simulink model "Complete_Model"  (R2023a)
settings: Solver=VariableStepAuto  StartTime=0.0  StopTime=22  MinStep=5e-5  RelTol=1e-3
values from neighbouring files (symbols this model uses):
  Cbus = 80e-3*n_wt_agg*u^2    (= 1.33333333333)    [param.m]
  D = 1e-3    (= 0.001)    [param.m]
  f = 50    [param.m]
  Fs = Vs * sqrt(2/3)/(2*pi*f)    [param.m]
  fsw = 4e3    (= 4000)    [param.m]
  Idr_rated = sqrt(2)*Is    [param.m]
  J = 127*n_wt_agg    (= 19050)    [param.m]
  ki_id = (wni^2)*Lr*sigma    (= 3.27733001332)    [param.m]
  ki_idg = (wnig^2)*Lg    (= 0.263189450696)    [param.m]
  ki_iq = ki_id    (= 3.27733001332)    [param.m]
  ki_iqg = ki_idg    (= 0.263189450696)    [param.m]
  ki_v = -300000*n_wt_agg    (= -45000000)    [param.m]
  Kopt = ((0.5*rho*pi*(Radius^5)*Cp_max)/(lambda_opt^3))    (= 296454.198186)    [param.m]
  kp_id = (2*wni*sigma*Lr) - Rr    (= 0.00384733333333)    [param.m]
  kp_idg = ((2*wnig*Lg)-Rg)    (= 0.00167538274858)    [param.m]
  kp_iq = kp_id    (= 0.00384733333333)    [param.m]
  kp_iqg = kp_idg    (= 0.00167538274858)    [param.m]
  kp_v = -1000*n_wt_agg    (= -150000)    [param.m]
  Kpg = 1/(1.5*Vr*sqrt(2/3))    [param.m]
  Kqg = -Kpg    [param.m]
  lambda_opt = 7.2    [param.m]
  Lg = 400e-6 /n_wt_agg    (= 2.66666666667e-06)    [param.m]
  Lm = 2.5e-3 /n_wt_agg    (= 1.66666666667e-05)    [param.m]
  Lr = Lm + Lsi    (= 1.72466666667e-05)    [param.m]
  Ls = Lm + Lsi    (= 1.72466666667e-05)    [param.m]
  Lsi = 0.087e-3 /n_wt_agg    (= 5.8e-07)    [param.m]
  N = 100    [param.m]
  n_wt_agg = 150    [param.m]
  p = 2    [param.m]
  Ps = 2e6*n_wt_agg    (= 300000000)    [param.m]
  Radius = 42    [param.m]
  Rg = 20e-6 /n_wt_agg    (= 1.33333333333e-07)    [param.m]
  rho = 1.225    [param.m]
  Rr = 2.9e-3 /n_wt_agg    (= 1.93333333333e-05)    [param.m]
  Rs = 2.6e-3 /n_wt_agg    (= 1.73333333333e-05)    [param.m]
  sigma = 1 - Lm^2 / (Ls*Lr)    (= 0.0661284179513)    [param.m]
  smax = 1/3    (= 0.333333333333)    [param.m]
  tab_lambda = [0.1:0.01:11.8]    [param.m]
  Vbus = 1150/u    (= 3450)    [param.m]
  Vs = 690    [param.m]
undefined symbols (not defined in the file or in its folder): Cp, D1, Delta, DiscIntNeverNeededParam, magnitude
libraries: spsSecondOrderFilterLib x133, spsThreePhaseVIMeasurementLib x58, spsPower3phInstantaneousLib x52, simulink x41, spsThreePhaseTransformerTwoWindingsLib x27, spsUniversalBridgeLib x22, spsPWMGenerator2LevelLib x22, spsSeriesRLCBranchLib x19, spsFirstOrderFilterLib x18, spsThreePhasePISectionLineLib x17, spsGroundLib x17, spsThreePhaseSeriesRLCBranchLib x17
(part 5 of 15)

== system Complete_Model/HVDC Link/Grid side control ==
  note: DC Voltage Control
  note: Reactive Power Control
  Vdc_mes : Inport
  Vdc_ref : Inport  Port=2
  Q_grid : Inport  Port=3
  Qref_grid : Inport  Port=4
  Clarke - Park Transform1 : eeTransforms/Park Transform  AxisAlignment=D-axis  PowerInvariant=off
      settings: LibrarySourceBlock=|
      | ee_sl_lib/Mathematical
      | Transforms/Park Transform
  Clarke - Park Transform2 : eeTransforms/Park Transform  AxisAlignment=D-axis  PowerInvariant=off
      settings: LibrarySourceBlock=|
      | ee_sl_lib/Mathematical
      | Transforms/Park Transform
  Clarke - Park Transform3 : eeTransforms/Park Transform  AxisAlignment=D-axis  PowerInvariant=off
      settings: LibrarySourceBlock=|
      | ee_sl_lib/Mathematical
      | Transforms/Park Transform
  Constant : Constant  Value=0
  Constant1 : Constant  Value=0
  Constant4 : Constant  Value=0
  Demux{1..5,7} (6 alike) : Demux  Outputs=2
      Demux2 settings: DisplayOption=none
      Demux3 settings: DisplayOption=none
  Display5 : Display
  Display6 : Display
  Divide : Product  Inputs=*/
  First-Order Filter{1,4..6} (4 alike) : spsFirstOrderFilterLib/First-Order Filter  FilterType=Lowpass  Tc=0.015915  Ts=7.4074e-05  Initialize=on  Vac_Init=[0, 0, 50]  Vdc_Init=1.2247  PlotResponse=off  FreqRange="[0,  200,  1]"
      settings: AttributesFormatString=Tc:%<Tc>\nIC:%<Vdc_Init>
  First-Order Filter2 : spsFirstOrderFilterLib/First-Order Filter  FilterType=Lowpass  Tc=0.015915*2  Ts=7.4074e-05  Initialize=on  Vac_Init=[0, 0, 50]  Vdc_Init=1.2247  PlotResponse=off  FreqRange="[0,  200,  1]"
      settings: AttributesFormatString=Tc:%<Tc>\nIC:%<Vdc_Init>
  First-Order Filter3 : spsFirstOrderFilterLib/First-Order Filter  FilterType=Lowpass  Tc=0.015915*2  Ts=7.4074e-05  Initialize=on  Vac_Init=[0, 0, 50]  Vdc_Init=1.2247  PlotResponse=off  FreqRange="[0,  200,  1]"
      settings: AttributesFormatString=Tc:%<Tc>\nIC:%<Vdc_Init>
  Gain : Gain  Gain=0.1947+0.178
  Gain1 : Gain  Gain=0.1947+0.178
  Gain2 : Gain  Gain=-1
  Gain4 : Gain  Gain=-1
  I_max : Constant  Value=1.1
  Inverse Clarke - Park Transform : eeTransforms/Inverse Park Transform  AxisAlignment=D-axis  PowerInvariant=off
      settings: LibrarySourceBlock=|
      | ee_sl_lib/Mathematical
      | Transforms/Inverse
      | Park Transform
  Math Function3 : Math  Operator=magnitude^2  SignedPower=on
  Math Function4 : Math  Operator=magnitude^2  SignedPower=on
  Math Function5 : Math  Operator=sqrt  SignedPower=on
  Mux : Mux  Inputs=3
  Mux1 : Mux  Inputs=2
  PID Controller : slpidlib/PID Controller  Controller=PID  Form=Parallel  TimeDomain=Continuous-time  UseExternalTs=off  SampleTime=-1  IntegratorMethod=Forward Euler  FilterMethod=Forward Euler  ControllerParametersSource=internal  P=0.6  I=6  UseKiTs=off  D=0  N=100  UseFilter=on  TunerSelectOption=Transfer Function Based (PID Tuner App)  InitialConditionSource=internal  InitialConditionForIntegrator=0  InitialConditionForFilter=0  DifferentiatorICPrevScaledInput=0  ExternalReset=none  TrackingMode=off  Kt=1  LimitOutput=on  SatLimitsSource=internal  UpperSaturationLimit=2  LowerSaturationLimit=-2  LinearizeAsGain=on  AntiWindupMode=clamping  Kb=1  LimitIntegrator=on  UpperIntegratorSaturationLimit=1.1  LowerIntegratorSaturationLimit=-1.1  POutMin=[]  POutMax=[]  IOutMin=[]  IOutMax=[]  DOutMin=[]  DOutMax=[]  NOutMin=[]  NOutMax=[]  SumOutMin=[]  SumOutMax=[]  SaturationOutMin=[]  SaturationOutMax=[]  PParamMin=[]  PParamMax=[]  IParamMin=[]  IParamMax=[]  DParamMin=[]  DParamMax=[]  NParamMin=[]  NParamMax=[]  KbParamMin=[]  KbParamMax=[]  KtParamMin=[]  KtParamMax=[]  KbOutMin=[]  KbOutMax=[]  KtOutMin=[]  KtOutMax=[]  IntegratorOutMin=[]  IntegratorOutMax=[]  FilterOutMin=[]  FilterOutMax=[]  SumI1OutMin=[]  SumI1OutMax=[]  SumI2OutMin=[]  SumI2OutMax=[]  SumI3OutMin=[]  SumI3OutMax=[]  SumI4OutMin=[]  SumI4OutMax=[]  SumDOutMin=[]  SumDOutMax=[]  DifferentiatorOutMin=[]  DifferentiatorOutMax=[]  FilterDiffOutCoefMin=[]  FilterDiffOutCoefMax=[]  ReciprocalOutMin=[]  ReciprocalOutMax=[]  SumDenOutMin=[]  SumDenOutMax=[]  SumNumOutMin=[]  SumNumOutMax=[]  DivideOutMin=[]  DivideOutMax=[]  UdiffTsProdOutMin=[]  UdiffTsProdOutMax=[]  NTsProdOutMin=[]  NTsProdOutMax=[]  UintegralTsProdOutMin=[]  UintegralTsProdOutMax=[]  UngainTsProdOutMin=[]  UngainTsProdOutMax=[]  ClampingZeroOutDataTypeStr=Inherit: Inherit via back propagation  ClampingZeroOutMin=[]  ClampingZeroOutMax=[]  FilterDenConstantOutDataTypeStr=Inherit: Inherit via back propagation  FilterDenConstantOutMin=[]  FilterDenConstantOutMax=[]  PCopyOutDataTypeStr=Inherit: Inherit via back propagation  PCopyOutMin=[]  PCopyOutMax=[]  NCopyOutDataTypeStr=Inherit: Inherit via back propagation  NCopyOutMin=[]  NCopyOutMax=[]  IntegratorContinuousStateAttributes=''  IntegratorStateMustResolveToSignalObject=off  IntegratorRTWStateStorageClass=Auto  FilterContinuousStateAttributes=''  FilterStateMustResolveToSignalObject=off  FilterRTWStateStorageClass=Auto  ParallelPVariant=InternalParameters  IdealPVariant=Disabled  IVariant=InternalParameters  DVariant=InternalParameters  IntegratorVariant=Continuous  SatVariant=Enabled  AWVariant=ContClampingParallel  PCopyVariant=Disabled  TRVariant=Disabled  FdbkBlocksVariant=Forward  IdealPFdbkVariant=Disabled  SatFdbkVariant=Disabled  DerivativeFilterVariant=ContFilter  NVariant=InternalParameters  NCopyVariant=Disabled  FilterICVariant=InternalICsFilter  IntegratorICVariant=InternalICs  ExternalResetVariant=Disabled  TRSumVariant=Passthrough  SumFdbkVariant=Disabled  SumVariant=Sum_PID  TsampFilterVariant=InternalTs  TsampNgainVariant=Passthrough  TsampIntegralVariant=Disabled_SignalSpec  ExternalSaturationOutDataTypeStr=Inherit: Same as second input
  PID Controller1 : slpidlib/PID Controller  Controller=PID  Form=Parallel  TimeDomain=Continuous-time  UseExternalTs=off  SampleTime=-1  IntegratorMethod=Forward Euler  FilterMethod=Forward Euler  ControllerParametersSource=internal  P=0.6  I=6  UseKiTs=off  D=0  N=100  UseFilter=on  TunerSelectOption=Transfer Function Based (PID Tuner App)  InitialConditionSource=internal  InitialConditionForIntegrator=0  InitialConditionForFilter=0  DifferentiatorICPrevScaledInput=0  ExternalReset=none  TrackingMode=off  Kt=1  LimitOutput=on  SatLimitsSource=internal  UpperSaturationLimit=2  LowerSaturationLimit=-2  LinearizeAsGain=on  AntiWindupMode=clamping  Kb=1  LimitIntegrator=on  UpperIntegratorSaturationLimit=1.1  LowerIntegratorSaturationLimit=-1.1  POutMin=[]  POutMax=[]  IOutMin=[]  IOutMax=[]  DOutMin=[]  DOutMax=[]  NOutMin=[]  NOutMax=[]  SumOutMin=[]  SumOutMax=[]  SaturationOutMin=[]  SaturationOutMax=[]  PParamMin=[]  PParamMax=[]  IParamMin=[]  IParamMax=[]  DParamMin=[]  DParamMax=[]  NParamMin=[]  NParamMax=[]  KbParamMin=[]  KbParamMax=[]  KtParamMin=[]  KtParamMax=[]  KbOutMin=[]  KbOutMax=[]  KtOutMin=[]  KtOutMax=[]  IntegratorOutMin=[]  IntegratorOutMax=[]  FilterOutMin=[]  FilterOutMax=[]  SumI1OutMin=[]  SumI1OutMax=[]  SumI2OutMin=[]  SumI2OutMax=[]  SumI3OutMin=[]  SumI3OutMax=[]  SumI4OutMin=[]  SumI4OutMax=[]  SumDOutMin=[]  SumDOutMax=[]  DifferentiatorOutMin=[]  DifferentiatorOutMax=[]  FilterDiffOutCoefMin=[]  FilterDiffOutCoefMax=[]  ReciprocalOutMin=[]  ReciprocalOutMax=[]  SumDenOutMin=[]  SumDenOutMax=[]  SumNumOutMin=[]  SumNumOutMax=[]  DivideOutMin=[]  DivideOutMax=[]  UdiffTsProdOutMin=[]  UdiffTsProdOutMax=[]  NTsProdOutMin=[]  NTsProdOutMax=[]  UintegralTsProdOutMin=[]  UintegralTsProdOutMax=[]  UngainTsProdOutMin=[]  UngainTsProdOutMax=[]  ClampingZeroOutDataTypeStr=Inherit: Inherit via back propagation  ClampingZeroOutMin=[]  ClampingZeroOutMax=[]  FilterDenConstantOutDataTypeStr=Inherit: Inherit via back propagation  FilterDenConstantOutMin=[]  FilterDenConstantOutMax=[]  PCopyOutDataTypeStr=Inherit: Inherit via back propagation  PCopyOutMin=[]  PCopyOutMax=[]  NCopyOutDataTypeStr=Inherit: Inherit via back propagation  NCopyOutMin=[]  NCopyOutMax=[]  IntegratorContinuousStateAttributes=''  IntegratorStateMustResolveToSignalObject=off  IntegratorRTWStateStorageClass=Auto  FilterContinuousStateAttributes=''  FilterStateMustResolveToSignalObject=off  FilterRTWStateStorageClass=Auto  ParallelPVariant=InternalParameters  IdealPVariant=Disabled  IVariant=InternalParameters  DVariant=InternalParameters  IntegratorVariant=Continuous  SatVariant=Enabled  AWVariant=ContClampingParallel  PCopyVariant=Disabled  TRVariant=Disabled  FdbkBlocksVariant=Forward  IdealPFdbkVariant=Disabled  SatFdbkVariant=Disabled  DerivativeFilterVariant=ContFilter  NVariant=InternalParameters  NCopyVariant=Disabled  FilterICVariant=InternalICsFilter  IntegratorICVariant=InternalICs  ExternalResetVariant=Disabled  TRSumVariant=Passthrough  SumFdbkVariant=Disabled  SumVariant=Sum_PID  TsampFilterVariant=InternalTs  TsampNgainVariant=Passthrough  TsampIntegralVariant=Disabled_SignalSpec  ExternalSaturationOutDataTypeStr=Inherit: Same as second input
  PID Controller2 : slpidlib/PID Controller  Controller=PI  Form=Parallel  TimeDomain=Continuous-time  UseExternalTs=off  SampleTime=7.4074e-05  IntegratorMethod=Forward Euler  FilterMethod=Forward Euler  ControllerParametersSource=internal  P=2  I=40  UseKiTs=off  D=0  N=100  UseFilter=on  TunerSelectOption=Transfer Function Based (PID Tuner App)  InitialConditionSource=internal  InitialConditionForIntegrator=0  InitialConditionForFilter=0  DifferentiatorICPrevScaledInput=0  ExternalReset=none  TrackingMode=off  Kt=1  LimitOutput=on  SatLimitsSource=internal  UpperSaturationLimit=1.1  LowerSaturationLimit=-1.1  LinearizeAsGain=on  AntiWindupMode=clamping  Kb=1  LimitIntegrator=on  UpperIntegratorSaturationLimit=20  LowerIntegratorSaturationLimit=-20  POutMin=[]  POutMax=[]  IOutMin=[]  IOutMax=[]  DOutMin=[]  DOutMax=[]  NOutMin=[]  NOutMax=[]  SumOutMin=[]  SumOutMax=[]  SaturationOutMin=[]  SaturationOutMax=[]  PParamMin=[]  PParamMax=[]  IParamMin=[]  IParamMax=[]  DParamMin=[]  DParamMax=[]  NParamMin=[]  NParamMax=[]  KbParamMin=[]  KbParamMax=[]  KtParamMin=[]  KtParamMax=[]  KbOutMin=[]  KbOutMax=[]  KtOutMin=[]  KtOutMax=[]  IntegratorOutMin=[]  IntegratorOutMax=[]  FilterOutMin=[]  FilterOutMax=[]  SumI1OutMin=[]  SumI1OutMax=[]  SumI2OutMin=[]  SumI2OutMax=[]  SumI3OutMin=[]  SumI3OutMax=[]  SumI4OutMin=[]  SumI4OutMax=[]  SumDOutMin=[]  SumDOutMax=[]  DifferentiatorOutMin=[]  DifferentiatorOutMax=[]  FilterDiffOutCoefMin=[]  FilterDiffOutCoefMax=[]  ReciprocalOutMin=[]  ReciprocalOutMax=[]  SumDenOutMin=[]  SumDenOutMax=[]  SumNumOutMin=[]  SumNumOutMax=[]  DivideOutMin=[]  DivideOutMax=[]  UdiffTsProdOutMin=[]  UdiffTsProdOutMax=[]  NTsProdOutMin=[]  NTsProdOutMax=[]  UintegralTsProdOutMin=[]  UintegralTsProdOutMax=[]  UngainTsProdOutMin=[]  UngainTsProdOutMax=[]  ClampingZeroOutDataTypeStr=Inherit: Inherit via back propagation  ClampingZeroOutMin=[]  ClampingZeroOutMax=[]  FilterDenConstantOutDataTypeStr=Inherit: Inherit via back propagation  FilterDenConstantOutMin=[]  FilterDenConstantOutMax=[]  PCopyOutDataTypeStr=Inherit: Inherit via back propagation  PCopyOutMin=[]  PCopyOutMax=[]  NCopyOutDataTypeStr=Inherit: Inherit via back propagation  NCopyOutMin=[]  NCopyOutMax=[]  IntegratorContinuousStateAttributes=''  IntegratorStateMustResolveToSignalObject=off  IntegratorRTWStateStorageClass=Auto  FilterContinuousStateAttributes=''  FilterStateMustResolveToSignalObject=off  FilterRTWStateStorageClass=Auto  ParallelPVariant=InternalParameters  IdealPVariant=Disabled  IVariant=InternalParameters  DVariant=Disabled  IntegratorVariant=Continuous  SatVariant=Enabled  AWVariant=ContClampingParallel  PCopyVariant=Disabled  TRVariant=Disabled  FdbkBlocksVariant=Forward  IdealPFdbkVariant=Disabled  SatFdbkVariant=Disabled  DerivativeFilterVariant=Disabled  NVariant=Disabled  NCopyVariant=Disabled_SignalSpec  FilterICVariant=Disabled  IntegratorICVariant=InternalICs  ExternalResetVariant=Disabled  TRSumVariant=Passthrough  SumFdbkVariant=Disabled  SumVariant=Sum_PI  TsampFilterVariant=InternalTs  TsampNgainVariant=Passthrough  TsampIntegralVariant=Disabled_SignalSpec  ExternalSaturationOutDataTypeStr=Inherit: Same as second input
  PID Controller5 : slpidlib/PID Controller  Controller=PI  Form=Parallel  TimeDomain=Continuous-time  UseExternalTs=off  SampleTime=-1  IntegratorMethod=Forward Euler  FilterMethod=Forward Euler  ControllerParametersSource=internal  P=2  I=4  UseKiTs=off  D=0  N=100  UseFilter=on  TunerSelectOption=Transfer Function Based (PID Tuner App)  InitialConditionSource=internal  InitialConditionForIntegrator=0  InitialConditionForFilter=0  DifferentiatorICPrevScaledInput=0  ExternalReset=none  TrackingMode=off  Kt=1  LimitOutput=on  SatLimitsSource=internal  UpperSaturationLimit=1.1  LowerSaturationLimit=-1.1  LinearizeAsGain=on  AntiWindupMode=clamping  Kb=1  LimitIntegrator=on  UpperIntegratorSaturationLimit=20  LowerIntegratorSaturationLimit=-20  POutMin=[]  POutMax=[]  IOutMin=[]  IOutMax=[]  DOutMin=[]  DOutMax=[]  NOutMin=[]  NOutMax=[]  SumOutMin=[]  SumOutMax=[]  SaturationOutMin=[]  SaturationOutMax=[]  PParamMin=[]  PParamMax=[]  IParamMin=[]  IParamMax=[]  DParamMin=[]  DParamMax=[]  NParamMin=[]  NParamMax=[]  KbParamMin=[]  KbParamMax=[]  KtParamMin=[]  KtParamMax=[]  KbOutMin=[]  KbOutMax=[]  KtOutMin=[]  KtOutMax=[]  IntegratorOutMin=[]  IntegratorOutMax=[]  FilterOutMin=[]  FilterOutMax=[]  SumI1OutMin=[]  SumI1OutMax=[]  SumI2OutMin=[]  SumI2OutMax=[]  SumI3OutMin=[]  SumI3OutMax=[]  SumI4OutMin=[]  SumI4OutMax=[]  SumDOutMin=[]  SumDOutMax=[]  DifferentiatorOutMin=[]  DifferentiatorOutMax=[]  FilterDiffOutCoefMin=[]  FilterDiffOutCoefMax=[]  ReciprocalOutMin=[]  ReciprocalOutMax=[]  SumDenOutMin=[]  SumDenOutMax=[]  SumNumOutMin=[]  SumNumOutMax=[]  DivideOutMin=[]  DivideOutMax=[]  UdiffTsProdOutMin=[]  UdiffTsProdOutMax=[]  NTsProdOutMin=[]  NTsProdOutMax=[]  UintegralTsProdOutMin=[]  UintegralTsProdOutMax=[]  UngainTsProdOutMin=[]  UngainTsProdOutMax=[]  ClampingZeroOutDataTypeStr=Inherit: Inherit via back propagation  ClampingZeroOutMin=[]  ClampingZeroOutMax=[]  FilterDenConstantOutDataTypeStr=Inherit: Inherit via back propagation  FilterDenConstantOutMin=[]  FilterDenConstantOutMax=[]  PCopyOutDataTypeStr=Inherit: Inherit via back propagation  PCopyOutMin=[]  PCopyOutMax=[]  NCopyOutDataTypeStr=Inherit: Inherit via back propagation  NCopyOutMin=[]  NCopyOutMax=[]  IntegratorContinuousStateAttributes=''  IntegratorStateMustResolveToSignalObject=off  IntegratorRTWStateStorageClass=Auto  FilterContinuousStateAttributes=''  FilterStateMustResolveToSignalObject=off  FilterRTWStateStorageClass=Auto  ParallelPVariant=InternalParameters  IdealPVariant=Disabled  IVariant=InternalParameters  DVariant=Disabled  IntegratorVariant=Continuous  SatVariant=Enabled  AWVariant=ContClampingParallel  PCopyVariant=Disabled  TRVariant=Disabled  FdbkBlocksVariant=Forward  IdealPFdbkVariant=Disabled  SatFdbkVariant=Disabled  DerivativeFilterVariant=Disabled  NVariant=Disabled  NCopyVariant=Disabled_SignalSpec  FilterICVariant=Disabled  IntegratorICVariant=InternalICs  ExternalResetVariant=Disabled  TRSumVariant=Passthrough  SumFdbkVariant=Disabled  SumVariant=Sum_PI  TsampFilterVariant=InternalTs  TsampNgainVariant=Passthrough  TsampIntegralVariant=Disabled_SignalSpec  ExternalSaturationOutDataTypeStr=Inherit: Same as second input
  Saturation Dynamic : simulink/Discontinuities/Saturation Dynamic  OutMin=[]  OutMax=[]  OutDataTypeStr=Inherit: Same as second input  OutDataType=fixdt(1, 16)  OutScaling=2^-10  DoSatur=off
  Saturation2 : Saturate  UpperLimit=1.1  LowerLimit=-1.1
  Scope13 : Scope  NumInputPorts=2
  Scope15 : Scope
  Selector1 : Selector  InputPortWidth=3  IndexOptions=Index vector (dialog)  Indices=[1 2]  OutputSizes=1
  Selector2 : Selector  InputPortWidth=3  IndexOptions=Index vector (dialog)  Indices=[1 2]  OutputSizes=1
  Selector3 : Selector  InputPortWidth=3  IndexOptions=Index vector (dialog)  Indices=[1 2]  OutputSizes=1
  Simple PLL : Trigonometry  Operator=atan2
  Step : Step  Time=0.5  Before=1  After=-1  SampleTime=0
  Step3 : Step  Time=0.05  Before=1  After=-1  SampleTime=0
  Sum : Sum  Inputs=|+-
  Sum10 : Sum  Inputs=++|
  Sum11 : Sum  Inputs=+-|
  Sum2 : Sum  Inputs=-+  SaturateOnIntegerOverflow=on
  Sum5 : Sum  Inputs=--+  InputSameDT=on  SaturateOnIntegerOverflow=on  OutDataTypeStr=Inherit: Same as first input
  Sum6 : Sum  Inputs=+|-|+  InputSameDT=on  SaturateOnIntegerOverflow=on  OutDataTypeStr=Inherit: Same as first input
  Sum7 : Sum  Inputs=|+-
  Sum8 : Sum  Inputs=|+-
  Switch : Switch  Criteria=u2 > Threshold  SaturateOnIntegerOverflow=off  InputSameDT=off
  Switch1 : Switch  Criteria=u2 > Threshold  SaturateOnIntegerOverflow=off  InputSameDT=off
  To File : ToFile  Filename=Iabc_grid_hvdc  SaveFormat=Timeseries
  To Workspace : ToWorkspace  VariableName=Egrid_d  SaveFormat=Timeseries  SampleTime=-1
  To Workspace1 : ToWorkspace  VariableName=Egrid_q  SaveFormat=Timeseries  SampleTime=-1
  To Workspace2 : ToWorkspace  VariableName=Igrid_q  SaveFormat=Timeseries  SampleTime=-1
  To Workspace3 : ToWorkspace  VariableName=Igrid_d  SaveFormat=Timeseries  SampleTime=-1
  To Workspace4 : ToWorkspace  VariableName=Igrid_d_ref  SaveFormat=Timeseries  SampleTime=-1
  To Workspace5 : ToWorkspace  VariableName=Igrid_q_ref  SaveFormat=Timeseries  SampleTime=-1
  To Workspace6 : ToWorkspace  VariableName=Uconv_grid_d  SaveFormat=Timeseries  SampleTime=-1
  To Workspace8 : ToWorkspace  VariableName=Uconv_grid_q  SaveFormat=Timeseries  SampleTime=-1
  ToFile_Egrid_d : ToFile  Filename=Egrid_d.mat  SaveFormat=Timeseries
  ToFile_Egrid_q : ToFile  Filename=Egrid_q.mat  SaveFormat=Timeseries
  ToFile_Igrid_d : ToFile  Filename=Igrid_d.mat  SaveFormat=Timeseries
  ToFile_Igrid_d_ref : ToFile  Filename=Igrid_d_ref.mat  SaveFormat=Timeseries
  ToFile_Igrid_q : ToFile  Filename=Igrid_q.mat  SaveFormat=Timeseries
  ToFile_Igrid_q_ref : ToFile  Filename=Igrid_q_ref.mat  SaveFormat=Timeseries
  ToFile_Uconv_grid_d : ToFile  Filename=Uconv_grid_d.mat  SaveFormat=Timeseries
  ToFile_Uconv_grid_q : ToFile  Filename=Uconv_grid_q.mat  SaveFormat=Timeseries
  abc->alpha/beta : Subsystem
  Vref_abc : Outport
  tag scope: Egrid_dq=global, Idmes_hvdc_BE=global, Idref_hvdc_BE=global, Igrid_dq=global, Iqmes_hvdc_BE=global, Iqref_hvdc_BE=global, Vdref_hvdc_BE=global, Vqref_hvdc_BE=global, theta_atan_grid=global
  wires:
  I_max/1 -> Math Function3/1
  Mux1/1 -> Demux3/1  "Idq_ref"
  Math Function3/1 -> Sum2/2
  Math Function5/1 -> Gain2/1, Saturation Dynamic/1
  Sum2/1 -> Math Function5/1
  Math Function4/1 -> Sum2/1
  [Iabc_grid] -> Clarke - Park Transform1/1, To File/1
  Clarke - Park Transform1/1 -> Selector1/1
  Demux1/2 -> To Workspace2/1, ToFile_Igrid_q/1, [Iqmes_hvdc_BE]
  Demux1/1 -> ToFile_Igrid_d/1, To Workspace3/1, [Idmes_hvdc_BE]
  Selector1/1 -> [Igrid_dq], Demux2/1, Demux1/1  "I_dq"
  Demux3/2 -> Sum8/1, Gain/1  "I_ref_q"
  Demux2/1 -> Sum7/2  "Iv_d"
  Demux2/2 -> Sum8/2  "Iv_q"
  PID Controller/1 -> Sum6/2
  Gain/1 -> Sum6/3
  [Egrid_q] -> Sum5/3
  Demux3/1 -> Sum7/1, Gain1/1  "I_ref_d"
  Gain1/1 -> Sum5/2
  Mux/1 -> Inverse Clarke - Park Transform/1
  Sum6/1 -> Mux/1, [Vdref_hvdc_BE]
  Sum5/1 -> Mux/2, [Vqref_hvdc_BE]
  [Egrid_d] -> Sum6/1
  Constant/1 -> Mux/3
  Gain2/1 -> Saturation Dynamic/3
  Saturation Dynamic/1 -> ToFile_Igrid_q_ref/1, [Iqref_hvdc_BE], Mux1/2, To Workspace5/1  "Igrid_q_ref"
  Vdc_mes/1 -> First-Order Filter2/1
  Sum/1 -> PID Controller2/1
  Vdc_ref/1 -> Switch1/3
  Step/1 -> Switch/2
  Constant1/1 -> Switch/1
  Sum7/1 -> PID Controller/1
  PID Controller1/1 -> Sum5/1
  Sum8/1 -> PID Controller1/1
  Simple PLL/1 -> Clarke - Park Transform2/2, [theta_atan_grid]  "theta_atan"
  abc->alpha/beta/1(alpha/beta) -> Demux4/1
  [Eabc_grid] -> Clarke - Park Transform2/1, abc->alpha/beta/1(abc)
  Demux4/2 -> Simple PLL/1
  Demux4/1 -> Simple PLL/2
  Selector2/1 -> Demux5/1, [Egrid_dq]  "E_dq"
  Clarke - Park Transform2/1 -> Selector2/1
  Demux5/2 -> First-Order Filter4/1, [Egrid_q]
  Demux5/1 -> First-Order Filter1/1, [Egrid_d]
  First-Order Filter2/1 -> Sum/2
  Inverse Clarke - Park Transform/1 -> Vref_abc/1
  Gain4/1 -> Switch/3
  Switch/1 -> Saturation Dynamic/2
  [Egrid_d] -> Divide/2
  PID Controller5/1 -> Sum10/2
  Q_grid/1 -> Sum11/2
  First-Order Filter3/1 -> Sum10/1
  Sum11/1 -> PID Controller5/1
  Divide/1 -> First-Order Filter3/1
  Sum10/1 -> Saturation2/1  "iqref"
  [theta_atan_grid] -> Inverse Clarke - Park Transform/2
  First-Order Filter1/1 -> To Workspace/1, ToFile_Egrid_d/1
  Saturation2/1 -> Gain4/1
  First-Order Filter6/1 -> To Workspace8/1, ToFile_Uconv_grid_q/1  "Uconv_grid_q"
  First-Order Filter5/1 -> To Workspace6/1, ToFile_Uconv_grid_d/1  "Uconv_grid_d"
  Demux7/1 -> Display5/1, Scope13/1, [Uconv_grid_d], First-Order Filter5/1
  Demux7/2 -> Scope13/2, Display6/1, [Uconv_grid_q], First-Order Filter6/1
  Clarke - Park Transform3/1 -> Selector3/1
  Selector3/1 -> Demux7/1  "U_dq"
  [Uconv_grid] -> Scope15/1, Clarke - Park Transform3/1
  PID Controller2/1 -> To Workspace4/1, ToFile_Igrid_d_ref/1, Mux1/1, [Idref_hvdc_BE], Math Function4/1
  [theta_atan_grid] -> Clarke - Park Transform1/2
  Qref_grid/1 -> Divide/1, Sum11/1
  Constant4/1 -> Switch1/1
  Step3/1 -> Switch1/2
  Switch1/1 -> Sum/1
  [theta_atan_grid] -> Clarke - Park Transform3/2
  First-Order Filter4/1 -> ToFile_Egrid_q/1, To Workspace1/1

== system Complete_Model/HVDC Link/Power compuations Grid Side ==
  note: Reactive Power Calculation
  note: Active Power Calculation
  Constant1 : Constant  Value=0
  Demux{,1..3} (4 alike) : Demux  Outputs=2
      settings: DisplayOption=none
  Product{,1..3} (4 alike) : Product  SaturateOnIntegerOverflow=on  OutDataTypeStr=Inherit: Same as first input
  Scope1 : Scope
  Scope2 : Scope
  Scope3 : Scope  NumInputPorts=2
  Scope4 : Scope
  Second-Order Low-Pass Filter 3 : spsSecondOrderFilterLib/Second-Order Filter  FilterType=Lowpass  Fo=17  Zeta=0.7  Ts=7.4074e-05  Initialize=off  Vac_Init="[0,  0,  60]"  Vdc_Init=0  PlotResponse=off  FreqRange=[0, 500, 1]
      settings: AttributesFormatString=f0=%<Fo>
  Second-Order Low-Pass Filter 4 : spsSecondOrderFilterLib/Second-Order Filter  FilterType=Lowpass  Fo=17  Zeta=0.7  Ts=7.4074e-05  Initialize=off  Vac_Init="[0,  0,  60]"  Vdc_Init=0  PlotResponse=off  FreqRange=[0, 500, 1]
      settings: AttributesFormatString=f0=%<Fo>
  Step1 : Step  Time=0.5  Before=1  After=-1  SampleTime=0
  Sum : Sum  SaturateOnIntegerOverflow=on
  Sum1 : Sum  Inputs=+-  SaturateOnIntegerOverflow=on
  Switch1 : Switch  Criteria=u2 > Threshold  SaturateOnIntegerOverflow=off  InputSameDT=off
  To Workspace1 : ToWorkspace  VariableName=Qgrid_ref  SaveFormat=Timeseries  SampleTime=-1
  To Workspace8 : ToWorkspace  VariableName=Pgrid_mes  SaveFormat=Timeseries  SampleTime=-1
  To Workspace9 : ToWorkspace  VariableName=Qgrid_mes  SaveFormat=Timeseries  SampleTime=-1
  ToFile_Pgrid_mes : ToFile  Filename=Pgrid_mes.mat  SaveFormat=Timeseries
  ToFile_Qgrid_mes : ToFile  Filename=Qgrid_mes.mat  SaveFormat=Timeseries
  ToFile_Qgrid_ref : ToFile  Filename=Qgrid_ref.mat  SaveFormat=Timeseries
  tag scope: P_hvdc_BE=global, Qmes_hvdc_BE=global
  wires:
  Demux1/1 -> Product/2
  Product1/1 -> Sum/2
  Demux/2 -> Product1/1
  Demux1/2 -> Product1/2
  Product/1 -> Sum/1
  Demux/1 -> Product/1
  Demux3/2 -> Product3/2
  Demux3/1 -> Product2/1
  Product2/1 -> Sum1/1
  [Igrid_dq] -> Demux3/1
  Demux2/2 -> Product2/2
  Product3/1 -> Sum1/2
  Sum/1 -> Scope1/1, Second-Order Low-Pass Filter 3/1
  [Egrid_dq] -> Demux/1
  [Igrid_dq] -> Demux1/1
  Second-Order Low-Pass Filter 4/1 -> ToFile_Qgrid_mes/1, [Qmes_hvdc_BE], To Workspace9/1, Scope4/1  "Qgrid_mes"
  [Egrid_dq] -> Demux2/1
  Second-Order Low-Pass Filter 3/1 -> ToFile_Pgrid_mes/1, To Workspace8/1, [P_hvdc_BE], Scope3/2  "Pgrid_mes"
  Demux2/1 -> Product3/1
  Sum1/1 -> Scope2/1, Second-Order Low-Pass Filter 4/1
  Step1/1 -> Switch1/2
  Switch1/1 -> To Workspace1/1, ToFile_Qgrid_ref/1  "Qgrid_ref"
  [Qref_grid] -> Switch1/3
  Constant1/1 -> Switch1/1

== system Complete_Model/HVDC Link/Power compuations Wind Farm Side ==
  note: Reactive Power Calculation
  note: Active Power Calculation
  Constant3 : Constant  Value=0
  Demux{,1..3} (4 alike) : Demux  Outputs=2
      settings: DisplayOption=none
  Product{,1..3} (4 alike) : Product  SaturateOnIntegerOverflow=on  OutDataTypeStr=Inherit: Same as first input
  Scope{1..2,4,37} (4 alike) : Scope
  Scope3 : Scope  NumInputPorts=2
  Second-Order Low-Pass Filter 1 : spsSecondOrderFilterLib/Second-Order Filter  FilterType=Lowpass  Fo=17  Zeta=0.7  Ts=7.4074e-05  Initialize=off  Vac_Init="[0,  0,  60]"  Vdc_Init=0  PlotResponse=off  FreqRange=[0, 500, 1]
      settings: AttributesFormatString=f0=%<Fo>
  Second-Order Low-Pass Filter 2 : spsSecondOrderFilterLib/Second-Order Filter  FilterType=Lowpass  Fo=17  Zeta=0.7  Ts=7.4074e-05  Initialize=off  Vac_Init="[0,  0,  60]"  Vdc_Init=0  PlotResponse=off  FreqRange=[0, 500, 1]
      settings: AttributesFormatString=f0=%<Fo>
  Step2 : Step  Time=0.5  Before=1  After=-1  SampleTime=0
  Sum : Sum  SaturateOnIntegerOverflow=on
  Sum1 : Sum  Inputs=+-  SaturateOnIntegerOverflow=on
  Switch3 : Switch  Criteria=u2 > Threshold  SaturateOnIntegerOverflow=off  InputSameDT=off
  To Workspace12 : ToWorkspace  VariableName=Qwind_ref  SaveFormat=Timeseries  SampleTime=-1
  To Workspace3 : ToWorkspace  VariableName=P_windfarm_mes  SaveFormat=Timeseries  SampleTime=-1
  To Workspace4 : ToWorkspace  VariableName=Q_windfarm_mes  SaveFormat=Timeseries  SampleTime=-1
  To Workspace5 : ToWorkspace  VariableName=P_windfarm_ref  SaveFormat=Timeseries  SampleTime=-1
  ToFile_P_windfarm_mes : ToFile  Filename=P_windfarm_mes.mat  SaveFormat=Timeseries
  ToFile_P_windfarm_ref : ToFile  Filename=P_windfarm_ref.mat  SaveFormat=Timeseries
  ToFile_Q_windfarm_mes : ToFile  Filename=Q_windfarm_mes.mat  SaveFormat=Timeseries
  ToFile_Qwind_ref : ToFile  Filename=Qwind_ref.mat  SaveFormat=Timeseries
  tag scope: P_hvdc_wfside=global, Q_hvdc_wfside=global
  wires:
  Switch3/1 -> ToFile_Qwind_ref/1, To Workspace12/1, Scope37/1  "Qwind_ref"
  [Qref_hvdc_wfside] -> Switch3/3
  Step2/1 -> Switch3/2
  Constant3/1 -> Switch3/1
  Sum/1 -> Scope1/1, Second-Order Low-Pass Filter 1/1
  Product/1 -> Sum/1
  Demux1/2 -> Product1/2
  Demux/1 -> Product/1
  Demux/2 -> Product1/1
  [Iwindfarm_dq] -> Demux1/1
  Demux1/1 -> Product/2
  Product1/1 -> Sum/2
  Demux3/2 -> Product3/2
  [Ewindfarm_dq] -> Demux2/1
  [Iwindfarm_dq] -> Demux3/1
  Product3/1 -> Sum1/2
  Product2/1 -> Sum1/1
  Demux3/1 -> Product2/1
  Sum1/1 -> Scope2/1, Second-Order Low-Pass Filter 2/1
  Demux2/2 -> Product2/2
  Demux2/1 -> Product3/1
  [Ewindfarm_dq] -> Demux/1
  Second-Order Low-Pass Filter 2/1 -> ToFile_Q_windfarm_mes/1, [Q_hvdc_wfside], To Workspace4/1, Scope4/1  "Q_windfarm_mes"
  [Pref_hvdc_wfside] -> To Workspace5/1, Scope3/1, ToFile_P_windfarm_ref/1  "P_windfarm_ref"
  Second-Order Low-Pass Filter 1/1 -> ToFile_P_windfarm_mes/1, [P_hvdc_wfside], To Workspace3/1, Scope3/2  "P_windfarm_mes"

== system Complete_Model/HVDC Link/Power losses & DC power ==
  Constant13 : Constant  Value=0.07+0.0072*45+0.07
  DC power1 : Scope
  Joule losses : Scope
  Product2 : Product
  Product3 : Product
  Product4 : Product
  Second-Order Filter5 : spsSecondOrderFilterLib/Second-Order Filter  FilterType=Lowpass  Fo=2000  Zeta=0.7  Ts=7.4074e-05  Initialize=off  Vac_Init="[0,  0,  60]"  Vdc_Init=0  PlotResponse=off  FreqRange=[0, 500, 1]
      settings: AttributesFormatString=f0=%<Fo>
  Square1 : Math  Operator=square
  Sum7 : Sum
  To Workspace7 : ToWorkspace  VariableName=Idc_mes_notpu  SaveFormat=Timeseries  SampleTime=-1
  ToFile_Idc_mes_notpu : ToFile  Filename=Idc_mes_notpu.mat  SaveFormat=Timeseries
  wires:
  Sum7/1 -> Second-Order Filter5/1
  [I_DC_neg] -> Product4/1
  Product2/1 -> Sum7/1
  Square1/1 -> Product3/2
  Constant13/1 -> Product3/1
  Second-Order Filter5/1 -> DC power1/1
  [VDC_neg_not_pu] -> Product4/2
  [I_DC] -> To Workspace7/1, Product2/1, ToFile_Idc_mes_notpu/1  "Idc_mes_notpu"
  Product3/1 -> Joule losses/1
  [VDC_not_pu] -> Product2/2
  [I_DC] -> Square1/1
  Product4/1 -> Sum7/2

== system Complete_Model/HVDC Link/Subsystem ==
  Constant1 : Constant  Commented=on  Value=0
  Constant4 : Constant  Commented=on  Value=0.2
  Constant8 : Constant  Value=0.2
  Divide : Product  Commented=on  Inputs=**
  From7 : From  Commented=on  GotoTag=Qwind_tot  TagVisibility=global
  Pulse Generator : DiscretePulseGenerator  Commented=on  PulseType=Time based  Period=3  PulseWidth=5
  Sample and Hold : spsSampleandHoldLib/Sample and Hold  Commented=on  ic=0  Ts=0
      settings: LibrarySourceBlock=|
      | sps_lib/Control/Sample and
      | Hold
  Second-Order Low-Pass Filter 1 : spsSecondOrderFilterLib/Second-Order Filter  Commented=on  FilterType=Lowpass  Fo=17  Zeta=0.7  Ts=7.4074e-05  Initialize=off  Vac_Init="[0,  0,  60]"  Vdc_Init=0  PlotResponse=off  FreqRange=[0, 500, 1]
      settings: AttributesFormatString=f0=%<Fo>
  Step : Step  Commented=on  Time=60  Before=1  After=-1  SampleTime=0
  Step1 : Step  Commented=on  Time=0.05  Before=1  After=-1  SampleTime=0
  Switch1 : Switch  Commented=on  Criteria=u2 > Threshold  SaturateOnIntegerOverflow=off  InputSameDT=off
  Zero-Order Hold1 : ZeroOrderHold  SampleTime=1/fsw
  in pu : Gain  Commented=on  Gain=1/2e9
  Qref_hvdc_wfside : Outport
  tag scope: Qref_hvdc_wfside=global
  wires:
  From7/1 -> in pu/1
  Second-Order Low-Pass Filter 1/1 -> Sample and Hold/1
  Pulse Generator/1 -> Sample and Hold/2
  Sample and Hold/1 -> ?
  Step/1 -> Divide/1
  in pu/1 -> Divide/2
  Divide/1 -> Second-Order Low-Pass Filter 1/1
  Step1/1 -> Switch1/2
  Constant1/1 -> Switch1/1
  Constant4/1 -> Switch1/3
  Constant8/1 -> Zero-Order Hold1/1
  Zero-Order Hold1/1 -> [Qref_hvdc_wfside], Qref_hvdc_wfside/1

== system Complete_Model/HVDC Link/Subsystem1 ==
  Constant3 : Constant  Value=0
  Constant8 : Constant  Value=0
  Scope : Scope
  Step2 : Step  Time=0.05  Before=1  After=-1  SampleTime=0
  Switch1 : Switch  Criteria=u2 > Threshold  SaturateOnIntegerOverflow=off  InputSameDT=off
  Qref_grid : Outport
  tag scope: Qref_hvdc_BE=global
  wires:
  Switch1/1 -> Qref_grid/1, [Qref_hvdc_BE], Scope/1
  Constant8/1 -> Switch1/3
  Constant3/1 -> Switch1/1
  Step2/1 -> Switch1/2

== system Complete_Model/HVDC Link/Subsystem7 ==
  note: add all windfarms
  Constant : Constant
  From : From  Commented=on  GotoTag=Pout
  From1 : From  Commented=on  GotoTag=Pout11  TagVisibility=global
  From2 : From  Commented=on  GotoTag=Pout22  TagVisibility=global
  From3 : From  Commented=on  GotoTag=Pout3  TagVisibility=global
  From4 : From  Commented=on  GotoTag=Pout7  TagVisibility=global
  From5 : From  Commented=on  GotoTag=Pout8  TagVisibility=global
  From6 : From  Commented=on  GotoTag=Pout4  TagVisibility=global
  Gain{,1..6} (7 alike) : Gain  Commented=on  Gain=-1
  If : If  IfExpression=u1 <= 1
  If Action Subsystem : Subsystem  TreatAsAtomicUnit=on
  If Action Subsystem 2 : Subsystem  TreatAsAtomicUnit=on
  Merge : Merge
  Pulse Generator1 : DiscretePulseGenerator  Commented=on  PulseType=Time based  Period=1  PulseWidth=5
  Sample and Hold1 : spsSampleandHoldLib/Sample and Hold  Commented=on  ic=0  Ts=0
      settings: LibrarySourceBlock=|
      | sps_lib/Control/Sample and
      | Hold
  Second-Order Low-Pass Filter 1 : spsSecondOrderFilterLib/Second-Order Filter  FilterType=Lowpass  Fo=17  Zeta=0.7  Ts=7.4074e-05  Initialize=off  Vac_Init="[0,  0,  60]"  Vdc_Init=0  PlotResponse=off  FreqRange=[0, 500, 1]
      settings: AttributesFormatString=f0=%<Fo>
  Sum : Sum  Commented=on  Inputs=|++
  Sum{1..5} (5 alike) : Sum  Commented=on  Inputs=++|
  in pu : Gain  Gain=1/2e9
  Pref_hvdc : Outport
  tag scope: Pref_hvdc_wfside=global
  wires:
  If Action Subsystem 2/1(Out1) -> Merge/2
  Constant/1 -> If Action Subsystem 2/1(In1)
  If/2 -> If Action Subsystem 2/Action
  If Action Subsystem/1(Pref_hvdc) -> Merge/1
  If/1 -> If Action Subsystem/Action
  From/1 -> Gain/1
  Gain/1 -> Sum/1
  From1/1 -> Gain1/1
  From2/1 -> Gain2/1
  From3/1 -> Gain3/1
  From4/1 -> Gain4/1
  Gain1/1 -> Sum1/1
  Gain3/1 -> Sum3/1
  Gain2/1 -> Sum2/1
  Sum3/1 -> Sum2/2
  Sum2/1 -> Sum1/2
  Sum1/1 -> Sum/2
  From5/1 -> Gain5/1
  Gain4/1 -> Sum4/1
  Sum4/1 -> Sum3/2
  Gain6/1 -> Sum5/2
  From6/1 -> Gain6/1
  Sum5/1 -> Sum4/2
  Gain5/1 -> Sum5/1
  in pu/1 -> Second-Order Low-Pass Filter 1/1
  [Pwind_tot] -> in pu/1
  Second-Order Low-Pass Filter 1/1 -> If Action Subsystem/1(In1), If/1
  ? -> Sample and Hold1/1
  Pulse Generator1/1 -> Sample and Hold1/2
  Merge/1 -> Pref_hvdc/1, [Pref_hvdc_wfside]

== system Complete_Model/HVDC Link/V_DC Measure ==
  In1 : Inport
  Scope27 : Scope
  Scope48 : Scope
  Scope8 : Scope  NumInputPorts=2
  Second-Order Filter2 : spsSecondOrderFilterLib/Second-Order Filter  FilterType=Lowpass  Fo=2000  Zeta=0.7  Ts=7.4074e-05  Initialize=off  Vac_Init="[0,  0,  60]"  Vdc_Init=0  PlotResponse=off  FreqRange=[0, 500, 1]
      settings: AttributesFormatString=f0=%<Fo>
  To Workspace2 : ToWorkspace  VariableName=Vdc_mes  SaveFormat=Timeseries  SampleTime=-1
  ToFile_Vdc_mes : ToFile  Filename=Vdc_mes.mat  SaveFormat=Timeseries
  in pu : Gain  Gain=1/(525e3)
  tag scope: VDC_not_pu=global, Vdcp_mes=global
  wires:
  Second-Order Filter2/1 -> ToFile_Vdc_mes/1, Scope27/1, [Vdcp_mes], To Workspace2/1  "Vdc_mes"
  in pu/1 -> Scope8/2, Second-Order Filter2/1
  [Vdc_ref] -> Scope8/1
  In1/1 -> [VDC_not_pu], in pu/1, Scope48/1

== system Complete_Model/HVDC Link/V_DC_neg Measure ==
  Scope49 : Scope
  Scope50 : Scope
  Voltage Measurement7 : spsVoltageMeasurementLib/Voltage Measurement  PhasorSimulation=off  OutputType=Complex  PSBequivalent=0
  pu5 : Gain  Gain=1/(525e3)
  + : PMIOPort  Side=Left
  - : PMIOPort  Port=2  Side=Left
  tag scope: VDC_neg_not_pu=global
  wires:
  Voltage Measurement7/1 -> [VDC_neg_not_pu], Scope50/1, pu5/1
  pu5/1 -> Scope49/1, [Vdcn_mes]
  connections:
  Voltage Measurement7/LConn1 -- +/RConn1
  Voltage Measurement7/LConn2 -- -/RConn1
